simulink model "TosAeb"  (R2021a)
settings: Solver=FixedStepDiscrete  StartTime=0.0  StopTime=inf  FixedStep=0.01  RelTol=1e-3
undefined symbols (not defined in the file or in its folder): ACCModSts, AccSts1, AEB_ObjectMovingOrientation, AEB_ObjectMovingState, CN_BTCActive, CN_CurrentGearR, CN_CurrentGearR_DHT, CN_PTCActive, CN_VDCActive, CN_VSC_EspOff, CN_VSC_FCW_AEB_Error, CN_VSC_FCW_AEB_NoDispay, CN_VSC_FCW_AEB_PerfmDgdtn, CN_VSC_FCW_AEB_TempUnAvlbl, CN_VSC_VehStandstill, CNaccelAvoidMax, CNaccelAvoidMin, CNbrakePedalPress, CNcomfortStrLeftAccelMax, CNcomfortStrLeftAccelMin, CNcomfortStrRightAccelMax, CNcomfortStrRightAccelMin, CNDrvDoorOpen, CNDrvModeEco, CNDrvModeNorm, CNDrvModeOther, CNDrvModeSport, CNDrvSBRreminder, CNNull, CNobjInvalidID, CNPassDoorOpen, CNPassSBRreminder, CNPEBSAccelNegInf, CNPEBSAccelNull, CNPEBSTimeInf, CNPEBSTimeNull, CNSTOPMAX, CNSTOPNULL, CNsubjWidth, CNSwtOFF, CNSwtON, CNTTCMAX, CNTTCNULL, CNVehConfigAEBCycNone, CNVehConfigAEBNone, CNVehConfigAEBPedNone, CNVehConfigFCWNone, CNVehConfigJANone, CollisionSeverity, FALSE, FCWSnvty, JACycObjCalcInfoDefaultParams, JAPedObjCalcInfoDefaultParams, JAVehObjCalcInfoDefaultParams, latCycObjCalcInfoDefaultParams, latPedObjCalcInfoDefaultParams, longObjCalcInfoDefaultParams, ModSts, movingStatus, ObjectType ... (+1112)
libraries: simulink x3031, AEBSFunctionLib x50, Ta_Library x33, AEBSStateMachineLib x23, Mylibrary x12, simulink_extras x7, ObjSelect_lib x3, dspstat3 x2
(part 39 of 102)

== system TosAeb/FcwAebJa_Step_sys/ALG_AEBSystem/ALG_AEBController/AEB_FuncCondCalcApp/JaPedFunctionCondCalc/AEBJAPedAEBfullSuppAborCond/AEBJAPedAEBfullSuppCondCalc/AEBJAPedAEBfullSuppVehCondCalc ==
  note: Change OR to AND
  subjVel : Inport
  VehConfigIbooster : Inport  Port=2
  ESP_MasterCylindBrakePress : Inport  Port=3
  AccrPedlPosnDiagc : Inport  Port=4
  SteerWheelAngle_Deg : Inport  Port=5
  LeftTurnSWSts : Inport  Port=6
  RightTurnSWSts : Inport  Port=7
  subjLatAccel : Inport  Port=8
  VSC_JAPedAEBfullSuppCond : Inport  Port=9
  Abs : Abs
  Abs1 : Abs
  Compare To Constant1 : simulink/Logic and Bit Operations/Compare To Constant  relop===  const=FALSE  OutDataTypeStr=boolean
  Compare To Constant3 : simulink/Logic and Bit Operations/Compare To Constant  relop=<  const=P_AEB_Func_JaPedAEBfullSubjLatAccelAbsSupThres  OutDataTypeStr=boolean
  Compare To Constant4 : simulink/Logic and Bit Operations/Compare To Constant  relop===  const=TRUE  OutDataTypeStr=boolean
  Compare To Constant5 : simulink/Logic and Bit Operations/Compare To Constant  relop===  const=FALSE  OutDataTypeStr=boolean
  Compare To Constant6 : simulink/Logic and Bit Operations/Compare To Constant  relop=>  const=P_AEB_Func_JaPedAEBfullGasPedalSupThres  OutDataTypeStr=boolean
  Compare To Constant7 : simulink/Logic and Bit Operations/Compare To Constant  relop===  const=TRUE  OutDataTypeStr=boolean
  Compare To Constant8 : simulink/Logic and Bit Operations/Compare To Constant  relop=>=  const=P_AEB_Func_JaPedAEBfullSubjVelMin  OutDataTypeStr=boolean
  Compare To Constant9 : simulink/Logic and Bit Operations/Compare To Constant  relop=<=  const=P_AEB_Func_JaPedAEBfullSubjVelMax  OutDataTypeStr=boolean
  Constant : Constant  Value=P_AEB_Func_JaPedAEBfullAccrCondAborDelayTime
  Constant1 : Constant  Value=Ts
  Constant2 : Constant  Value=P_AEB_Func_JaPedAEBfullTurnSwtStsConfig
  Constant3 : Constant  Value=TRUE
  Logical Operator : Logic  AllPortsSameDT=off  OutDataTypeStr=boolean
  Logical Operator1 : Logic  Operator=OR  AllPortsSameDT=off  OutDataTypeStr=boolean
  Logical Operator2 : Logic  AllPortsSameDT=off  OutDataTypeStr=boolean
  Logical Operator3 : Logic  Operator=OR  AllPortsSameDT=off  OutDataTypeStr=boolean
  Logical Operator5 : Logic  Inputs=6  AllPortsSameDT=off  OutDataTypeStr=boolean
  Relational Operator : RelationalOperator  OutDataTypeStr=boolean  RndMeth=Simplest
  Relational Operator1 : RelationalOperator  Operator=<=  OutDataTypeStr=boolean  RndMeth=Simplest
  StateDelayAbortion1 : AEBSFunctionLib/StateDelayAbortion  SystemSampleTime=-1  VariantActivationTime=update diagram  Latency=0  AutoFrameSizeCalculation=off
  SteerWheelThresMax : Lookup_n-D  NumberOfTableDimensions=1  BreakpointsForDimension1=P_AEB_Func_JaPedAEBfullSubjVelStrWheelAngleMaxThresX  BeginIndexSearchUsingPreviousIndexResult=on  Table=P_AEB_Func_JaPedAEBfullSubjVelStrWheelAngleMaxThresY  InternalRulePriority=Speed  RndMeth=Simplest  InputSameDT=on
  SteerWheelThresMin Table : Lookup_n-D  NumberOfTableDimensions=1  BreakpointsForDimension1=P_AEB_Func_JaPedAEBfullSubjVelStrWheelAngleMinThresX  BeginIndexSearchUsingPreviousIndexResult=on  Table=P_AEB_Func_JaPedAEBfullSubjVelStrWheelAngleMinThresY  InternalRulePriority=Speed  RndMeth=Simplest  InputSameDT=on
  Switch : Switch  Criteria=u2 ~= 0
  brakeReleventSuppCond : Subsystem
  AEBJAPedAEBfullVehActiveCondFulfilled : Outport
  wires:
  VehConfigIbooster/1 -> brakeReleventSuppCond/1(VehConfigIbooster)
  Constant3/1 -> Switch/3
  Constant2/1 -> Switch/2
  Logical Operator1/1 -> Switch/1
  ESP_MasterCylindBrakePress/1 -> brakeReleventSuppCond/2(ESP_MasterCylindBrakePress)
  Compare To Constant5/1 -> Logical Operator5/2  "DriverReleventActiveCondFulfilled"
  Logical Operator3/1 -> Compare To Constant5/1
  Logical Operator2/1 -> Logical Operator5/3  "strReleventActiveCondFulfilled"
  Relational Operator/1 -> Logical Operator2/1
  SteerWheelThresMax/1 -> Relational Operator1/2
  [subjVel] -> SteerWheelThresMin Table/1, SteerWheelThresMax/1
  Relational Operator1/1 -> Logical Operator2/2
  Abs/1 -> Relational Operator/1, Relational Operator1/1
  Compare To Constant4/1 -> Logical Operator1/2
  Compare To Constant7/1 -> Logical Operator1/1
  RightTurnSWSts/1 -> Compare To Constant4/1
  Compare To Constant3/1 -> Logical Operator5/5  "LaterAccReleventActiveCondFulfilled"
  Abs1/1 -> Compare To Constant3/1
  subjLatAccel/1 -> Abs1/1
  SteerWheelThresMin Table/1 -> Relational Operator/2
  Constant1/1 -> StateDelayAbortion1/3
  Constant/1 -> StateDelayAbortion1/2
  Compare To Constant6/1 -> StateDelayAbortion1/1
  Logical Operator5/1 -> AEBJAPedAEBfullVehActiveCondFulfilled/1  "AEBJAPedAEBfullVehActiveCondFulfilled"
  subjVel/1 -> Compare To Constant8/1, [subjVel], Compare To Constant9/1
  Logical Operator/1 -> Logical Operator5/1  "VelocityReleventActiveCondFulfilled"
  Switch/1 -> Logical Operator5/4  "TrunSWReleventActiveCondFulfilled"
  AccrPedlPosnDiagc/1 -> Compare To Constant6/1
  Compare To Constant9/1 -> Logical Operator/2
  brakeReleventSuppCond/1(brakeReleventSuppCond) -> Logical Operator3/1  "BrkReleventSuppCondFulfilled"
  SteerWheelAngle_Deg/1 -> Abs/1
  Compare To Constant8/1 -> Logical Operator/1
  LeftTurnSWSts/1 -> Compare To Constant7/1
  StateDelayAbortion1/1 -> Logical Operator3/2  "accReleventSuppCondFulfilled"
  VSC_JAPedAEBfullSuppCond/1 -> Compare To Constant1/1
  Compare To Constant1/1 -> Logical Operator5/6

== system TosAeb/FcwAebJa_Step_sys/ALG_AEBSystem/ALG_AEBController/AEB_FuncCondCalcApp/JaPedFunctionCondCalc/AEBJAPedPrefillSuppAborCond/AEBJAPedPrefillAborCondCalc/AEBJAPedPrefillAborCondFinalJudge ==
  AEBJAPedPrefillObjRelAborCondFulfilled : Inport
  AEBJAPedPrefillVehRelAborCondFulfilled : Inport  Port=2
  AEBJAPedPrefillSituationRelAborCondFulfilled : Inport  Port=3
  Compare To Constant1 : simulink/Logic and Bit Operations/Compare To Constant  relop===  const=TRUE  OutDataTypeStr=boolean
  Compare To Constant2 : simulink/Logic and Bit Operations/Compare To Constant  relop===  const=TRUE  OutDataTypeStr=boolean
  Compare To Constant3 : simulink/Logic and Bit Operations/Compare To Constant  relop===  const=TRUE  OutDataTypeStr=boolean
  Logical Operator : Logic  Operator=OR  Inputs=3  AllPortsSameDT=off  OutDataTypeStr=boolean
  AEBJAPedPrefillAborCondFulfilled : Outport
  wires:
  Compare To Constant3/1 -> Logical Operator/1
  AEBJAPedPrefillVehRelAborCondFulfilled/1 -> Compare To Constant1/1
  AEBJAPedPrefillSituationRelAborCondFulfilled/1 -> Compare To Constant2/1
  Logical Operator/1 -> AEBJAPedPrefillAborCondFulfilled/1  "AEBJAPedPrefillAborCondFulfilled"
  AEBJAPedPrefillObjRelAborCondFulfilled/1 -> Compare To Constant3/1
  Compare To Constant1/1 -> Logical Operator/2
  Compare To Constant2/1 -> Logical Operator/3

== system TosAeb/FcwAebJa_Step_sys/ALG_AEBSystem/ALG_AEBController/AEB_FuncCondCalcApp/JaPedFunctionCondCalc/AEBJAPedPrefillSuppAborCond/AEBJAPedPrefillAborCondCalc/AEBJAPedPrefillObjRelAborCondCalc ==
  ObjectID : Inport
  Compare To Constant4 : simulink/Logic and Bit Operations/Compare To Constant  relop===  const=CNobjInvalidID  OutDataTypeStr=boolean
  Logical Operator : Logic  Operator=OR  AllPortsSameDT=off  OutDataTypeStr=boolean
  Relational Operator2 : RelationalOperator  Operator=~=  OutDataTypeStr=boolean  RndMeth=Simplest
  Unit Delay1 : UnitDelay  SampleTime=-1  HasFrameUpgradeWarning=on
  AEBJAPedPrefillObjRelAborCondFulfilled : Outport
  wires:
  ObjectID/1 -> Unit Delay1/1, Relational Operator2/1, Compare To Constant4/1
  Logical Operator/1 -> AEBJAPedPrefillObjRelAborCondFulfilled/1
  Unit Delay1/1 -> Relational Operator2/2
  Compare To Constant4/1 -> Logical Operator/1
  Relational Operator2/1 -> Logical Operator/2

== system TosAeb/FcwAebJa_Step_sys/ALG_AEBSystem/ALG_AEBController/AEB_FuncCondCalcApp/JaPedFunctionCondCalc/AEBJAPedPrefillSuppAborCond/AEBJAPedPrefillAborCondCalc/AEBJAPedPrefillSituationRelAborCondCalc ==
  Constant : Constant  Value=FALSE
  AEBJAPedPrefillSituationRelAborCondFulfilled : Outport
  wires:
  Constant/1 -> AEBJAPedPrefillSituationRelAborCondFulfilled/1

== system TosAeb/FcwAebJa_Step_sys/ALG_AEBSystem/ALG_AEBController/AEB_FuncCondCalcApp/JaPedFunctionCondCalc/AEBJAPedPrefillSuppAborCond/AEBJAPedPrefillAborCondCalc/AEBJAPedPrefillVehRelAborCondCalc ==
  SteerWheelSpd_DegS : Inport
  AccelPedalPositionDiag : Inport  Port=2
  VSC_JAPedPrefillAborCond : Inport  Port=3
  Abs : Abs
  Abs1 : Abs
  Compare To Constant : simulink/Logic and Bit Operations/Compare To Constant  relop=>  const=P_AEB_Func_JaPedPrefillStrWheelAngleSpdAborThres  OutDataTypeStr=boolean
  Compare To Constant1 : simulink/Logic and Bit Operations/Compare To Constant  relop=>  const=P_AEB_Func_JaPedPrefillGasPedalAborThres  OutDataTypeStr=boolean
  Compare To Constant2 : simulink/Logic and Bit Operations/Compare To Constant  relop===  const=TRUE  OutDataTypeStr=boolean
  Logical Operator2 : Logic  Operator=OR  Inputs=3  AllPortsSameDT=off  OutDataTypeStr=boolean
  AEBJAPedPrefillVehRelAborCondFulfilled : Outport
  wires:
  Compare To Constant2/1 -> Logical Operator2/3
  VSC_JAPedPrefillAborCond/1 -> Compare To Constant2/1
  Logical Operator2/1 -> AEBJAPedPrefillVehRelAborCondFulfilled/1  "AEBJAPedPrefillVehRelAborCondFulfilled"
  SteerWheelSpd_DegS/1 -> Abs/1
  Abs1/1 -> Compare To Constant1/1
  AccelPedalPositionDiag/1 -> Abs1/1
  Abs/1 -> Compare To Constant/1
  Compare To Constant/1 -> Logical Operator2/1
  Compare To Constant1/1 -> Logical Operator2/2

== system TosAeb/FcwAebJa_Step_sys/ALG_AEBSystem/ALG_AEBController/AEB_FuncCondCalcApp/JaPedFunctionCondCalc/AEBJAPedPrefillSuppAborCond/AEBJAPedPrefillSuppCondCalc/AEBJAPedPrefillSuppCondFinalJudge ==
  AEBJAPedPrefillObjRelActiveCondFulfilled : Inport
  AEBJAPedPrefillVehRelActiveCondFulfilled : Inport  Port=2
  AEBJAPedPrefillSituationRelActiveCondFulfilled : Inport  Port=3
  Compare To Constant : simulink/Logic and Bit Operations/Compare To Constant  relop===  const=FALSE  OutDataTypeStr=boolean
  Compare To Constant1 : simulink/Logic and Bit Operations/Compare To Constant  relop===  const=FALSE  OutDataTypeStr=boolean
  Compare To Constant2 : simulink/Logic and Bit Operations/Compare To Constant  relop===  const=FALSE  OutDataTypeStr=boolean
  Logical Operator : Logic  Operator=OR  Inputs=3  AllPortsSameDT=off  OutDataTypeStr=boolean
  AEBJAPedPrefillSuppCondFulfilled : Outport
  wires:
  Compare To Constant2/1 -> Logical Operator/3
  Compare To Constant1/1 -> Logical Operator/2
  Compare To Constant/1 -> Logical Operator/1
  AEBJAPedPrefillVehRelActiveCondFulfilled/1 -> Compare To Constant1/1
  AEBJAPedPrefillObjRelActiveCondFulfilled/1 -> Compare To Constant/1
  AEBJAPedPrefillSituationRelActiveCondFulfilled/1 -> Compare To Constant2/1
  Logical Operator/1 -> AEBJAPedPrefillSuppCondFulfilled/1

== system TosAeb/FcwAebJa_Step_sys/ALG_AEBSystem/ALG_AEBController/AEB_FuncCondCalcApp/JaPedFunctionCondCalc/AEBJAPedPrefillSuppAborCond/AEBJAPedPrefillSuppCondCalc/JaPedPrefillSuppSituationCondCalc ==
  distBraking : Inport
  distRadical2Collsion : Inport  Port=2
  tPredictTime : Inport  Port=3
  TTB : Inport  Port=4
  ObjColliFlg : Inport  Port=5
  Add : Sum  Inputs=2
  Add1 : Sum  Inputs=2
  Compare To Constant : simulink/Logic and Bit Operations/Compare To Constant  relop===  const=TRUE  OutDataTypeStr=boolean
  Constant2 : Constant  Value=P_AEB_Func_JaPedPrefillBrkDistSecure
  Constant3 : Constant  Value=P_AEB_Func_JaPedPrefillTtcTimeOffset
  Logical Operator1 : Logic  AllPortsSameDT=off  OutDataTypeStr=boolean
  Relational Operator : RelationalOperator  Operator=>  OutDataTypeStr=boolean  RndMeth=Simplest
  Relational Operator1 : RelationalOperator  Operator=<  OutDataTypeStr=boolean  RndMeth=Simplest
  Terminator : Terminator
  AEBJAVehPrefillSuppSitActiveCondFulfilled : Outport
  wires:
  TTB/1 -> Add1/1
  tPredictTime/1 -> Relational Operator1/1
  distRadical2Collsion/1 -> Relational Operator/2
  Add/1 -> Relational Operator/1
  distBraking/1 -> Add/1
  Constant2/1 -> Add/2
  Relational Operator1/1 -> Logical Operator1/1
  Logical Operator1/1 -> AEBJAVehPrefillSuppSitActiveCondFulfilled/1
  Relational Operator/1 -> Terminator/1
  Constant3/1 -> Add1/2
  Add1/1 -> Relational Operator1/2
  ObjColliFlg/1 -> Compare To Constant/1
  Compare To Constant/1 -> Logical Operator1/2

== system TosAeb/FcwAebJa_Step_sys/ALG_AEBSystem/ALG_AEBController/AEB_FuncCondCalcApp/JaPedFunctionCondCalc/AEBJAPedPrefillSuppAborCond/AEBJAPedPrefillSuppCondCalc/JaPedPrefillSuppSubjCondCalcu ==
  note: Chang OR to AND
  note: Change < to <=,change > to >=
  subjVel : Inport
  VehConfigIbooster : Inport  Port=2
  BrakePedalStatus_G : Inport  Port=3
  ESP_MasterCylindBrakePress : Inport  Port=4
  IB_BrkPedalAppld : Inport  Port=5
  AccrPedlPosnDiagc : Inport  Port=6
  SteerWheelAngle_Deg : Inport  Port=7
  LeftTurnSWSts : Inport  Port=8
  RightTurnSWSts : Inport  Port=9
  subjLatAccel : Inport  Port=10
  VSC_JAPedPrefillSuppCond : Inport  Port=11
  1-D Lookup Table : Lookup_n-D  NumberOfTableDimensions=1  BreakpointsForDimension1=P_AEB_Func_JaPedPrefillSubjVelStrWheelAngleMinThresX  BeginIndexSearchUsingPreviousIndexResult=on  Table=P_AEB_Func_JaPedPrefillSubjVelStrWheelAngleMinThresY  ExtrapMethod=Clip  InternalRulePriority=Speed  RndMeth=Simplest  InputSameDT=on
  1-D Lookup Table1 : Lookup_n-D  NumberOfTableDimensions=1  BreakpointsForDimension1=P_AEB_Func_JaPedPrefillSubjVelStrWheelAngleMaxThresX  BeginIndexSearchUsingPreviousIndexResult=on  Table=P_AEB_Func_JaPedPrefillSubjVelStrWheelAngleMaxThresY  ExtrapMethod=Clip  InternalRulePriority=Speed  RndMeth=Simplest  InputSameDT=on
  Abs : Abs
  Abs1 : Abs
  Compare To Constant2 : simulink/Logic and Bit Operations/Compare To Constant  relop===  const=FALSE  OutDataTypeStr=boolean
  Compare To Constant3 : simulink/Logic and Bit Operations/Compare To Constant  relop=<  const=P_AEB_Func_JaPedPrefillSubjLatAccelAbsSupThres  OutDataTypeStr=boolean
  Compare To Constant4 : simulink/Logic and Bit Operations/Compare To Constant  relop===  const=FALSE  OutDataTypeStr=boolean
  Compare To Constant5 : simulink/Logic and Bit Operations/Compare To Constant  relop===  const=TRUE  OutDataTypeStr=boolean
  Compare To Constant6 : simulink/Logic and Bit Operations/Compare To Constant  relop=>  const=P_AEB_Func_JaPedPrefillGasPedalSupThres  OutDataTypeStr=boolean
  Compare To Constant7 : simulink/Logic and Bit Operations/Compare To Constant  relop===  const=TRUE  OutDataTypeStr=boolean
  Compare To Constant8 : simulink/Logic and Bit Operations/Compare To Constant  relop=>=  const=P_AEB_Func_JaPedPrefillSubjVelMin  OutDataTypeStr=boolean
  Compare To Constant9 : simulink/Logic and Bit Operations/Compare To Constant  relop=<=  const=P_AEB_Func_JaPedPrefillSubjVelMax  OutDataTypeStr=boolean
  Constant : Constant  Value=P_AEB_Func_JaPedPrefillAccrCondAborDelayTime
  Constant1 : Constant  Value=Ts
  Constant2 : Constant  Value=P_AEB_Func_JaPedPrefillTurnSwtStsConfig
  Constant3 : Constant  Value=TRUE
  Logical Operator : Logic  AllPortsSameDT=off  OutDataTypeStr=boolean
  Logical Operator1 : Logic  Operator=OR  AllPortsSameDT=off  OutDataTypeStr=boolean
  Logical Operator2 : Logic  AllPortsSameDT=off  OutDataTypeStr=boolean
  Logical Operator4 : Logic  Operator=OR  AllPortsSameDT=off  OutDataTypeStr=boolean
  Logical Operator5 : Logic  Inputs=6  AllPortsSameDT=off  OutDataTypeStr=boolean
  Relational Operator : RelationalOperator  OutDataTypeStr=boolean  RndMeth=Simplest
  Relational Operator1 : RelationalOperator  Operator=<=  OutDataTypeStr=boolean  RndMeth=Simplest
  StateDelayAbortion : AEBSFunctionLib/StateDelayAbortion  SystemSampleTime=-1  VariantActivationTime=update diagram  Latency=0  AutoFrameSizeCalculation=off
  Switch : Switch  Criteria=u2 ~= 0
  brakeReleventSuppCond : Subsystem
  AEBJAPedPrefillVehActiveCondFulfilled : Outport
  wires:
  BrakePedalStatus_G/1 -> brakeReleventSuppCond/2(BrakePedalStatus_G)
  ESP_MasterCylindBrakePress/1 -> brakeReleventSuppCond/3(ESP_MasterCylindBrakePress)
  VehConfigIbooster/1 -> brakeReleventSuppCond/1(VehConfigIbooster)
  Logical Operator1/1 -> Switch/1
  Constant2/1 -> Switch/2
  Constant3/1 -> Switch/3
  IB_BrkPedalAppld/1 -> brakeReleventSuppCond/4(IB_BrkPedalAppld)
  Compare To Constant2/1 -> Logical Operator5/2
  Logical Operator4/1 -> Compare To Constant2/1
  Relational Operator1/1 -> Logical Operator2/2
  1-D Lookup Table1/1 -> Relational Operator1/2
  Relational Operator/1 -> Logical Operator2/1
  Compare To Constant5/1 -> Logical Operator1/2
  Compare To Constant7/1 -> Logical Operator1/1
  RightTurnSWSts/1 -> Compare To Constant5/1
  LeftTurnSWSts/1 -> Compare To Constant7/1
  Compare To Constant8/1 -> Logical Operator/1
  Logical Operator2/1 -> Logical Operator5/3  "strReleventActivepCondFulfilled"
  brakeReleventSuppCond/1(brakeReleventSuppCond) -> Logical Operator4/1  "BrkReleventSuppCondFulfilled"
  Compare To Constant9/1 -> Logical Operator/2
  AccrPedlPosnDiagc/1 -> Compare To Constant6/1
  Switch/1 -> Logical Operator5/4  "TrunSWReleventActiveCondFulfilled"
  Logical Operator/1 -> Logical Operator5/1  "VelocityReleventActiveCondFulfilled"
  subjVel/1 -> [subjVel], Compare To Constant8/1, Compare To Constant9/1
  Logical Operator5/1 -> AEBJAPedPrefillVehActiveCondFulfilled/1  "AEBJAPedPrefillVehActiveCondFulfilled"
  Constant/1 -> StateDelayAbortion/2
  Constant1/1 -> StateDelayAbortion/3
  [subjVel] -> 1-D Lookup Table/1, 1-D Lookup Table1/1
  1-D Lookup Table/1 -> Relational Operator/2
  subjLatAccel/1 -> Abs1/1
  Abs1/1 -> Compare To Constant3/1
  Compare To Constant3/1 -> Logical Operator5/5  "LaterAccReleventActiveCondFulfilled"
  VSC_JAPedPrefillSuppCond/1 -> Compare To Constant4/1
  Compare To Constant4/1 -> Logical Operator5/6  "VSCReleventActiveCondFulfilled"
  SteerWheelAngle_Deg/1 -> Abs/1
  Abs/1 -> Relational Operator1/1, Relational Operator/1
  Compare To Constant6/1 -> StateDelayAbortion/1
  StateDelayAbortion/1 -> Logical Operator4/2  "accReleventSuppCondFulfilled"

== system TosAeb/FcwAebJa_Step_sys/ALG_AEBSystem/ALG_AEBController/AEB_FuncCondCalcApp/JaPedFunctionCondCalc/AEBJAPedPrefillSuppAborCond/AEBJAPedPrefillSuppCondCalc/JaPedhPrefillSuppObjCondCalcu ==
  ObjectMovingOrientation : Inport
  ObjectID : Inport  Port=2
  ObjectExistProb : Inport  Port=3
  ObjectIsObstacleProb : Inport  Port=4
  ObjectTypeCameraConfirmed : Inport  Port=5
  dy : Inport  Port=6
  vxAbsObj : Inport  Port=7
  vyRelCorr : Inport  Port=8
  ObjFusQuality : Inport  Port=9
  Abs : Abs
  Compare To Constant1 : simulink/Logic and Bit Operations/Compare To Constant  relop=>  const=P_AEB_Func_JaPedPrefillObjVelSupThresMin  OutDataTypeStr=boolean
  Compare To Constant11 : simulink/Logic and Bit Operations/Compare To Constant  relop===  const=TRUE  OutDataTypeStr=boolean
  Compare To Constant15 : simulink/Logic and Bit Operations/Compare To Constant  relop=~=  const=CNobjInvalidID  OutDataTypeStr=boolean
  Compare To Constant16 : simulink/Logic and Bit Operations/Compare To Constant  relop=>  const=P_AEB_Func_JaPedPrefillObjObstacleProbSupThres  OutDataTypeStr=boolean
  Compare To Constant17 : simulink/Logic and Bit Operations/Compare To Constant  relop=~=  const=CNobjInvalidID  OutDataTypeStr=boolean
  Compare To Constant18 : simulink/Logic and Bit Operations/Compare To Constant  relop===  const=TRUE  OutDataTypeStr=boolean
  Compare To Constant2 : simulink/Logic and Bit Operations/Compare To Constant  relop=<  const=P_AEB_Func_JaPedPrefillObjVelSupThresMax  OutDataTypeStr=boolean
  Compare To Constant3 : simulink/Logic and Bit Operations/Compare To Constant  relop===  const=AEB_ObjectMovingOrientation.Crossing  OutDataTypeStr=boolean
  Compare To Constant4 : simulink/Logic and Bit Operations/Compare To Constant  relop===  const=AEB_ObjectMovingOrientation.InvDir  OutDataTypeStr=boolean
  Compare To Constant5 : simulink/Logic and Bit Operations/Compare To Constant  relop=<  const=P_AEB_Func_JaPedPrefillObjDySupThres  OutDataTypeStr=boolean
  Logical Operator1 : Logic  AllPortsSameDT=off  OutDataTypeStr=boolean
  Logical Operator2 : Logic  Operator=OR  AllPortsSameDT=off  OutDataTypeStr=boolean
  Logical Operator4 : Logic  Inputs=7  AllPortsSameDT=off  OutDataTypeStr=boolean
  Logical Operator6 : Logic  AllPortsSameDT=off  OutDataTypeStr=boolean
  Logical Operator7 : Logic  AllPortsSameDT=off  OutDataTypeStr=boolean
  Math Function : Math  Operator=hypot  SignedPower=on  SaturateOnIntegerOverflow=on
  Product1 : Product
  Relational Operator2 : RelationalOperator  Operator===  OutDataTypeStr=boolean  RndMeth=Simplest
  Unit Delay1 : UnitDelay  SampleTime=-1  HasFrameUpgradeWarning=on
  AEBJAPedPrefillSuppObjActiveCondFulfilled : Outport
  wires:
  Compare To Constant11/1 -> Logical Operator4/7  "ObjFusQuality"
  ObjFusQuality/1 -> Compare To Constant11/1
  Compare To Constant5/1 -> Logical Operator4/5
  Abs/1 -> Compare To Constant5/1
  dy/1 -> Abs/1
  Compare To Constant18/1 -> Logical Operator4/4  "objFusionCondFulfilled"
  Logical Operator2/1 -> Logical Operator4/1  "objMovingOrientationCondFulfilled"
  Logical Operator6/1 -> Logical Operator4/2  "objIDCondFulfilled"
  Logical Operator7/1 -> Logical Operator4/3  "objQualityCondFulfilled"
  Logical Operator1/1 -> Logical Operator4/6  "objVelocityCondFulfilled"
  Compare To Constant3/1 -> Logical Operator2/2
  Compare To Constant4/1 -> Logical Operator2/1
  ObjectMovingOrientation/1 -> Compare To Constant4/1, Compare To Constant3/1
  Compare To Constant15/1 -> Logical Operator6/1
  Relational Operator2/1 -> Logical Operator6/2
  Unit Delay1/1 -> Relational Operator2/2
  ObjectID/1 -> Compare To Constant17/1, Unit Delay1/1, Relational Operator2/1, Compare To Constant15/1
  ObjectExistProb/1 -> Product1/1
  Product1/1 -> Compare To Constant16/1
  ObjectIsObstacleProb/1 -> Product1/2
  ObjectTypeCameraConfirmed/1 -> Compare To Constant18/1
  vxAbsObj/1 -> Math Function/1
  vyRelCorr/1 -> Math Function/2
  Math Function/1 -> Compare To Constant1/1, Compare To Constant2/1
  Compare To Constant1/1 -> Logical Operator1/1
  Compare To Constant2/1 -> Logical Operator1/2
  Logical Operator4/1 -> AEBJAPedPrefillSuppObjActiveCondFulfilled/1
  Compare To Constant17/1 -> Logical Operator7/1
  Compare To Constant16/1 -> Logical Operator7/2

== system TosAeb/FcwAebJa_Step_sys/ALG_AEBSystem/ALG_AEBController/AEB_FuncCondCalcApp/JaVehFunctionCondCalc/AEBJAVehAEBfullSuppAborCond/AEBJAVehAEBfullAborCondCalc/AEBJAVehAEBfullAborCondFinalJudge ==
  AEBJAVehAEBfullObjRelAborCondFulfilled : Inport
  AEBJAVehAEBfullVehRelAborCondFulfilled : Inport  Port=2
  Compare To Constant1 : simulink/Logic and Bit Operations/Compare To Constant  relop===  const=TRUE  OutDataTypeStr=boolean
  Compare To Constant3 : simulink/Logic and Bit Operations/Compare To Constant  relop===  const=TRUE  OutDataTypeStr=boolean
  Logical Operator : Logic  Operator=OR  AllPortsSameDT=off  OutDataTypeStr=boolean
  AEBJAVehAEBfullAborCondNoStndstillFulfilled : Outport
  AEBJAVehAEBfullAborCondStndstillFulfilled : Outport  Port=2
  wires:
  Compare To Constant3/1 -> Logical Operator/1
  AEBJAVehAEBfullVehRelAborCondFulfilled/1 -> Compare To Constant1/1
  Logical Operator/1 -> AEBJAVehAEBfullAborCondNoStndstillFulfilled/1  "AEBJAVehAEBfullAborCondNoStndstillFulfilled"
  AEBJAVehAEBfullObjRelAborCondFulfilled/1 -> Compare To Constant3/1
  Compare To Constant1/1 -> Logical Operator/2, AEBJAVehAEBfullAborCondStndstillFulfilled/1  "AEBJAVehAEBfullAborCondStndstillFulfilled"

== system TosAeb/FcwAebJa_Step_sys/ALG_AEBSystem/ALG_AEBController/AEB_FuncCondCalcApp/JaVehFunctionCondCalc/AEBJAVehAEBfullSuppAborCond/AEBJAVehAEBfullAborCondCalc/AEBJAVehAEBfullObjRelAborCondCalc ==
  ObjectID : Inport
  Compare To Constant4 : simulink/Logic and Bit Operations/Compare To Constant  relop===  const=CNobjInvalidID  OutDataTypeStr=boolean
  Logical Operator : Logic  Operator=OR  AllPortsSameDT=off  OutDataTypeStr=boolean
  Relational Operator2 : RelationalOperator  Operator=~=  OutDataTypeStr=boolean  RndMeth=Simplest
  Unit Delay1 : UnitDelay  SampleTime=-1  HasFrameUpgradeWarning=on
  AEBJAVehAEBfullObjRelAborCondFulfilled : Outport
  wires:
  Logical Operator/1 -> AEBJAVehAEBfullObjRelAborCondFulfilled/1
  ObjectID/1 -> Unit Delay1/1, Relational Operator2/1, Compare To Constant4/1
  Unit Delay1/1 -> Relational Operator2/2
  Compare To Constant4/1 -> Logical Operator/1
  Relational Operator2/1 -> Logical Operator/2

== system TosAeb/FcwAebJa_Step_sys/ALG_AEBSystem/ALG_AEBController/AEB_FuncCondCalcApp/JaVehFunctionCondCalc/AEBJAVehAEBfullSuppAborCond/AEBJAVehAEBfullAborCondCalc/AEBJAVehAEBfullVehRelAborCondCalc ==
  SteerWheelAngleSign : Inport
  SteerWheelSpd_DegS : Inport  Port=2
  AccelPedalPositionDiag : Inport  Port=3
  VSC_JAVehAEBfullAborCond : Inport  Port=4
  Abs : Abs
  Abs1 : Abs
  Compare To Constant : simulink/Logic and Bit Operations/Compare To Constant  relop=>  const=P_AEB_Func_JaVehAEBfullStrWheelAngleSpdAborThres  OutDataTypeStr=boolean
  Compare To Constant1 : simulink/Logic and Bit Operations/Compare To Constant  relop=>  const=P_AEB_Func_JaVehAEBfullGasPedalAborThres  OutDataTypeStr=boolean
  Compare To Constant2 : simulink/Logic and Bit Operations/Compare To Constant  relop===  const=TRUE  OutDataTypeStr=boolean
  Compare To Constant3 : simulink/Logic and Bit Operations/Compare To Constant  relop===  const=TRUE  OutDataTypeStr=boolean
  Logical Operator2 : Logic  Operator=OR  Inputs=4  AllPortsSameDT=off  OutDataTypeStr=boolean
  AEBJAVehAEBfullVehRelAborCondFulfilled : Outport
  wires:
  Compare To Constant3/1 -> Logical Operator2/4
  VSC_JAVehAEBfullAborCond/1 -> Compare To Constant3/1
  Logical Operator2/1 -> AEBJAVehAEBfullVehRelAborCondFulfilled/1  "AEBJAVehAEBfullVehRelAborCondFulfilled"
  SteerWheelSpd_DegS/1 -> Abs/1
  Abs1/1 -> Compare To Constant1/1
  AccelPedalPositionDiag/1 -> Abs1/1
  Abs/1 -> Compare To Constant/1
  SteerWheelAngleSign/1 -> Compare To Constant2/1
  Compare To Constant2/1 -> Logical Operator2/1
  Compare To Constant/1 -> Logical Operator2/2
  Compare To Constant1/1 -> Logical Operator2/3

== system TosAeb/FcwAebJa_Step_sys/ALG_AEBSystem/ALG_AEBController/AEB_FuncCondCalcApp/JaVehFunctionCondCalc/AEBJAVehAEBfullSuppAborCond/AEBJAVehAEBfullSuppCondCalc/AEBJAVehAEBfullSuppCondFinalJudge ==
  AEBJAVehAEBfullObjRelActiveCondFulfilled : Inport
  AEBJAVehAEBfullVehRelActiveCondFulfilled : Inport  Port=2
  AEBJAVehAEBfullSituationRelActiveCondFulfilled : Inport  Port=3
  Compare To Constant : simulink/Logic and Bit Operations/Compare To Constant  relop===  const=FALSE  OutDataTypeStr=boolean
  Compare To Constant1 : simulink/Logic and Bit Operations/Compare To Constant  relop===  const=FALSE  OutDataTypeStr=boolean
  Compare To Constant2 : simulink/Logic and Bit Operations/Compare To Constant  relop===  const=FALSE  OutDataTypeStr=boolean
  Logical Operator : Logic  Operator=OR  Inputs=3  AllPortsSameDT=off  OutDataTypeStr=boolean
  AEBJAVehAEBfullSuppCondFulfilled : Outport
  wires:
  Compare To Constant2/1 -> Logical Operator/3
  Compare To Constant1/1 -> Logical Operator/2
  Compare To Constant/1 -> Logical Operator/1
  AEBJAVehAEBfullVehRelActiveCondFulfilled/1 -> Compare To Constant1/1
  AEBJAVehAEBfullObjRelActiveCondFulfilled/1 -> Compare To Constant/1
  AEBJAVehAEBfullSituationRelActiveCondFulfilled/1 -> Compare To Constant2/1
  Logical Operator/1 -> AEBJAVehAEBfullSuppCondFulfilled/1

== system TosAeb/FcwAebJa_Step_sys/ALG_AEBSystem/ALG_AEBController/AEB_FuncCondCalcApp/JaVehFunctionCondCalc/AEBJAVehAEBfullSuppAborCond/AEBJAVehAEBfullSuppCondCalc/AEBJAVehAEBfullSuppObjCondCalcu ==
  ObjectMovingState : Inport
  ObjectMovingOrientation : Inport  Port=2
  ObjectID : Inport  Port=3
  ObjectExistProb : Inport  Port=4
  ObjectIsObstacleProb : Inport  Port=5
  ObjectTypeCameraConfirmed : Inport  Port=6
  dy : Inport  Port=7
  vxAbsObj : Inport  Port=8
  vyRelCorr : Inport  Port=9
  ObjFusQuality : Inport  Port=10
  Compare To Constant1 : simulink/Logic and Bit Operations/Compare To Constant  relop=>  const=P_AEB_Func_JaVehAEBfullObjVelSupThresMin  OutDataTypeStr=boolean
  Compare To Constant11 : simulink/Logic and Bit Operations/Compare To Constant  relop===  const=TRUE  OutDataTypeStr=boolean
  Compare To Constant14 : simulink/Logic and Bit Operations/Compare To Constant  relop===  const=AEB_ObjectMovingState.Moving  OutDataTypeStr=boolean
  Compare To Constant15 : simulink/Logic and Bit Operations/Compare To Constant  relop=~=  const=CNobjInvalidID  OutDataTypeStr=boolean
  Compare To Constant16 : simulink/Logic and Bit Operations/Compare To Constant  relop=>  const=P_AEB_Func_JaVehAEBfullObjObstacleProbSupThres  OutDataTypeStr=boolean
  Compare To Constant17 : simulink/Logic and Bit Operations/Compare To Constant  relop=~=  const=CNobjInvalidID  OutDataTypeStr=boolean
  Compare To Constant18 : simulink/Logic and Bit Operations/Compare To Constant  relop===  const=TRUE  OutDataTypeStr=boolean
  Compare To Constant2 : simulink/Logic and Bit Operations/Compare To Constant  relop=<  const=P_AEB_Func_JaVehAEBfullObjVelSupThresMax  OutDataTypeStr=boolean
  Compare To Constant3 : simulink/Logic and Bit Operations/Compare To Constant  relop=>  const=P_AEB_Func_JaVehAEBfullObjDySupThresMin  OutDataTypeStr=boolean
  Compare To Constant4 : simulink/Logic and Bit Operations/Compare To Constant  relop===  const=AEB_ObjectMovingOrientation.InvDir  OutDataTypeStr=boolean
  Compare To Constant5 : simulink/Logic and Bit Operations/Compare To Constant  relop=<  const=P_AEB_Func_JaVehAEBfullObjDySupThresMax  OutDataTypeStr=boolean
  Logical Operator{1..2,6..7} (4 alike) : Logic  AllPortsSameDT=off  OutDataTypeStr=boolean
  Logical Operator4 : Logic  Inputs=8  AllPortsSameDT=off  OutDataTypeStr=boolean
  Math Function : Math  Operator=hypot  SignedPower=on  SaturateOnIntegerOverflow=on
  Product1 : Product
  Relational Operator2 : RelationalOperator  Operator===  OutDataTypeStr=boolean  RndMeth=Simplest
  Unit Delay1 : UnitDelay  SampleTime=-1  HasFrameUpgradeWarning=on
  AEBJAVehAEBfullObjActiveCondFulfilled : Outport
  wires:
  Compare To Constant11/1 -> Logical Operator4/8  "ObjFusQuality"
  ObjFusQuality/1 -> Compare To Constant11/1
  Logical Operator2/1 -> Logical Operator4/6  "objVelocityCondFulfilled"
  Compare To Constant5/1 -> Logical Operator2/2
  Compare To Constant3/1 -> Logical Operator2/1
  dy/1 -> Compare To Constant3/1, Compare To Constant5/1
  ObjectMovingState/1 -> Compare To Constant14/1
  Compare To Constant14/1 -> Logical Operator4/1  "objMovingStateCondFulfilled"
  ObjectMovingOrientation/1 -> Compare To Constant4/1
  Compare To Constant4/1 -> Logical Operator4/2  "objMovingOrientationCondFulfilled"
  Compare To Constant15/1 -> Logical Operator6/1
  Logical Operator6/1 -> Logical Operator4/3  "objIDCondFulfilled"
  Relational Operator2/1 -> Logical Operator6/2
  Unit Delay1/1 -> Relational Operator2/2
  ObjectID/1 -> Unit Delay1/1, Compare To Constant17/1, Compare To Constant15/1, Relational Operator2/1
  ObjectExistProb/1 -> Product1/1
  Product1/1 -> Compare To Constant16/1
  ObjectIsObstacleProb/1 -> Product1/2
  Compare To Constant16/1 -> Logical Operator7/2
  Compare To Constant17/1 -> Logical Operator7/1
  Logical Operator7/1 -> Logical Operator4/4  "objQualityCondFulfilled"
  ObjectTypeCameraConfirmed/1 -> Compare To Constant18/1
  Compare To Constant18/1 -> Logical Operator4/5  "objFusionCondFulfilled"
  vxAbsObj/1 -> Math Function/1
  vyRelCorr/1 -> Math Function/2
  Math Function/1 -> Compare To Constant1/1, Compare To Constant2/1
  Compare To Constant1/1 -> Logical Operator1/1
  Compare To Constant2/1 -> Logical Operator1/2
  Logical Operator1/1 -> Logical Operator4/7  "objVelocityCondFulfilled"
  Logical Operator4/1 -> AEBJAVehAEBfullObjActiveCondFulfilled/1

== system TosAeb/FcwAebJa_Step_sys/ALG_AEBSystem/ALG_AEBController/AEB_FuncCondCalcApp/JaVehFunctionCondCalc/AEBJAVehAEBfullSuppAborCond/AEBJAVehAEBfullSuppCondCalc/AEBJAVehAEBfullSuppSituationCondCalc ==
  note: change ">" to "<"
  initialLatDist : Inport
  restLatDist2Collsion : Inport  Port=2
  tPredictTime : Inport  Port=3
  TTB : Inport  Port=4
  ObjColliFlg : Inport  Port=5
  Add : Sum  Inputs=2
  Add1 : Sum  Inputs=2
  Add2 : Sum  Inputs=2
  Compare To Constant : simulink/Logic and Bit Operations/Compare To Constant  relop===  const=TRUE  OutDataTypeStr=boolean
  Constant : Constant  Value=P_Global_VehicleWidthMin_m
  Constant1 : Constant  Value=P_AEB_Func_JaVehAEBfullObjWidth
  Constant2 : Constant  Value=P_AEB_Func_JaVehAEBfullBrkDistSecure
  Constant3 : Constant  Value=P_AEB_Func_JaVehAEBfullTtcTimeOffset
  Gain : Gain  Gain=0.5
  Gain1 : Gain  Gain=0.5
  Logical Operator : Logic  AllPortsSameDT=off  OutDataTypeStr=boolean
  Logical Operator2 : Logic  AllPortsSameDT=off  OutDataTypeStr=boolean
  Relational Operator : RelationalOperator  Operator=>  OutDataTypeStr=boolean  RndMeth=Simplest
  Relational Operator1 : RelationalOperator  Operator=<=  OutDataTypeStr=boolean  RndMeth=Simplest
  Relational Operator2 : RelationalOperator  Operator=<  OutDataTypeStr=boolean  RndMeth=Simplest
  Terminator : Terminator
  AEBJAVehAEBfullActiveSituationCondFulfilled : Outport
  wires:
  Compare To Constant/1 -> Logical Operator2/2
  restLatDist2Collsion/1 -> Relational Operator1/1
  Relational Operator1/1 -> Logical Operator/2
  Relational Operator/1 -> Logical Operator/1
  Add/1 -> Add1/1
  Relational Operator2/1 -> Logical Operator2/1
  Constant3/1 -> Add2/2
  Add2/1 -> Relational Operator2/2
  ObjColliFlg/1 -> Compare To Constant/1
  tPredictTime/1 -> Relational Operator2/1
  TTB/1 -> Add2/1
  initialLatDist/1 -> Relational Operator/1
  Add1/1 -> Relational Operator/2, Relational Operator1/2
  Constant/1 -> Gain/1
  Gain/1 -> Add/1
  Constant1/1 -> Gain1/1
  Gain1/1 -> Add/2
  Constant2/1 -> Add1/2
  Logical Operator2/1 -> AEBJAVehAEBfullActiveSituationCondFulfilled/1
  Logical Operator/1 -> Terminator/1

== system TosAeb/FcwAebJa_Step_sys/ALG_AEBSystem/ALG_AEBController/AEB_FuncCondCalcApp/JaVehFunctionCondCalc/AEBJAVehAEBfullSuppAborCond/AEBJAVehAEBfullSuppCondCalc/AEBJAVehAEBfullSuppSubjCondCalcu ==
  note: Change or to and
  note: Change or to and
  subjVel : Inport
  VehConfigIbooster : Inport  Port=2
  ESP_MasterCylindBrakePress : Inport  Port=3
  AccrPedlPosnDiagc : Inport  Port=4
  SteerWheelAngle_Deg : Inport  Port=5
  LeftTurnSWSts : Inport  Port=6
  LateralAcc : Inport  Port=7
  VSC_JAVehAEBfullSuppCond : Inport  Port=8
  1-D Lookup Table : Lookup_n-D  NumberOfTableDimensions=1  BreakpointsForDimension1=P_AEB_Func_JaVehAEBfullSubjVelStrWheelAngleMinThresX  BeginIndexSearchUsingPreviousIndexResult=on  Table=P_AEB_Func_JaVehAEBfullSubjVelStrWheelAngleMinThresY  InternalRulePriority=Speed  RndMeth=Simplest  InputSameDT=on
  1-D Lookup Table1 : Lookup_n-D  NumberOfTableDimensions=1  BreakpointsForDimension1=P_AEB_Func_JaVehAEBfullSubjVelStrWheelAngleMaxThresX  BeginIndexSearchUsingPreviousIndexResult=on  Table=P_AEB_Func_JaVehAEBfullSubjVelStrWheelAngleMaxThresY  InternalRulePriority=Speed  RndMeth=Simplest  InputSameDT=on
  Abs : Abs
  Abs1 : Abs
  Compare To Constant1 : simulink/Logic and Bit Operations/Compare To Constant  relop===  const=FALSE  OutDataTypeStr=boolean
  Compare To Constant2 : simulink/Logic and Bit Operations/Compare To Constant  relop===  const=FALSE  OutDataTypeStr=boolean
  Compare To Constant3 : simulink/Logic and Bit Operations/Compare To Constant  relop=<=  const=P_AEB_Func_JaVehAEBfullSubjLatAccelAbsSupThres  OutDataTypeStr=boolean
  Compare To Constant6 : simulink/Logic and Bit Operations/Compare To Constant  relop=>  const=P_AEB_Func_JaVehAEBfullGasPedalSupThres  OutDataTypeStr=boolean
  Compare To Constant7 : simulink/Logic and Bit Operations/Compare To Constant  relop===  const=TRUE  OutDataTypeStr=boolean
  Compare To Constant8 : simulink/Logic and Bit Operations/Compare To Constant  relop=>=  const=P_AEB_Func_JaVehAEBfullSubjVelMin  OutDataTypeStr=boolean
  Compare To Constant9 : simulink/Logic and Bit Operations/Compare To Constant  relop=<=  const=P_AEB_Func_JaVehAEBfullSubjVelMax  OutDataTypeStr=boolean
  Constant : Constant  Value=P_AEB_Func_JaVehAEBfullAccrCondAborDelayTime
  Constant1 : Constant  Value=Ts
  Constant2 : Constant  Value=P_AEB_Func_JaVehAEBfullTurnSwtStsConfig
  Constant3 : Constant  Value=TRUE
  Logical Operator : Logic  AllPortsSameDT=off  OutDataTypeStr=boolean
  Logical Operator1 : Logic  Operator=OR  AllPortsSameDT=off  OutDataTypeStr=boolean
  Logical Operator4 : Logic  AllPortsSameDT=off  OutDataTypeStr=boolean
  Logical Operator5 : Logic  Inputs=6  AllPortsSameDT=off  OutDataTypeStr=boolean
  Relational Operator : RelationalOperator  Operator=>  OutDataTypeStr=boolean  RndMeth=Simplest
  Relational Operator1 : RelationalOperator  Operator=<  OutDataTypeStr=boolean  RndMeth=Simplest
  StateDelayAbortion1 : AEBSFunctionLib/StateDelayAbortion  SystemSampleTime=-1  VariantActivationTime=update diagram  Latency=0  AutoFrameSizeCalculation=off
  Switch : Switch  Criteria=u2 ~= 0
  brakeReleventSuppCond : Subsystem
  AEBJAVehAEBfullVehActiveCondFulfilled : Outport
  wires:
  VehConfigIbooster/1 -> brakeReleventSuppCond/1(VehConfigIbooster)
  ESP_MasterCylindBrakePress/1 -> brakeReleventSuppCond/2(ESP_MasterCylindBrakePress)
  Compare To Constant7/1 -> Switch/1
  Constant3/1 -> Switch/3
  Constant2/1 -> Switch/2
  Logical Operator1/1 -> Compare To Constant2/1
  Compare To Constant2/1 -> Logical Operator5/2  "DriverCondFulfiiled"
  1-D Lookup Table1/1 -> Relational Operator1/2
  Relational Operator1/1 -> Logical Operator4/1
  Compare To Constant1/1 -> Logical Operator5/6  "VSC_JAVehAEBfullActiveCond"
  VSC_JAVehAEBfullSuppCond/1 -> Compare To Constant1/1
  StateDelayAbortion1/1 -> Logical Operator1/2  "accReleventSuppCondFulfilled"
  LeftTurnSWSts/1 -> Compare To Constant7/1
  Compare To Constant8/1 -> Logical Operator/1
  Abs/1 -> Relational Operator1/1, Relational Operator/1
  SteerWheelAngle_Deg/1 -> Abs/1
  Logical Operator4/1 -> Logical Operator5/3  "strReleventActiveCondFulfilled"
  brakeReleventSuppCond/1(brakeReleventSuppCond) -> Logical Operator1/1  "BrkReleventSuppCondFulfilled"
  Compare To Constant9/1 -> Logical Operator/2
  AccrPedlPosnDiagc/1 -> Compare To Constant6/1
  Switch/1 -> Logical Operator5/4  "LeftTrunSWReleventActiveCondFulfilled"
  Logical Operator/1 -> Logical Operator5/1  "VelocityReleventActiveCondFulfilled"
  subjVel/1 -> Compare To Constant9/1, Compare To Constant8/1, [subjVel]
  Logical Operator5/1 -> AEBJAVehAEBfullVehActiveCondFulfilled/1  "AEBJAVehAEBfullVehActiveCondFulfilled"
  Compare To Constant6/1 -> StateDelayAbortion1/1
  Constant/1 -> StateDelayAbortion1/2
  Constant1/1 -> StateDelayAbortion1/3
  Relational Operator/1 -> Logical Operator4/2
  [subjVel] -> 1-D Lookup Table1/1, 1-D Lookup Table/1
  1-D Lookup Table/1 -> Relational Operator/2
  LateralAcc/1 -> Abs1/1
  Abs1/1 -> Compare To Constant3/1
  Compare To Constant3/1 -> Logical Operator5/5  "VSC_JAVehAEBfullActiveCond"

== system TosAeb/FcwAebJa_Step_sys/ALG_AEBSystem/ALG_AEBController/AEB_FuncCondCalcApp/JaVehFunctionCondCalc/AEBJAVehPrefillSuppAborCond/AEBJAVehPrefillAborCondCalc/AEBJAVehPrefillObjRelAborCondCalc ==
  ObjectID : Inport
  Compare To Constant4 : simulink/Logic and Bit Operations/Compare To Constant  relop===  const=CNobjInvalidID  OutDataTypeStr=boolean
  Logical Operator : Logic  Operator=OR  AllPortsSameDT=off  OutDataTypeStr=boolean
  Relational Operator2 : RelationalOperator  Operator=~=  OutDataTypeStr=boolean  RndMeth=Simplest
  Unit Delay1 : UnitDelay  SampleTime=-1  HasFrameUpgradeWarning=on
  AEBJAVehPrefillObjRelAborCondFulfilled : Outport
  wires:
  Relational Operator2/1 -> Logical Operator/2
  Compare To Constant4/1 -> Logical Operator/1
  Unit Delay1/1 -> Relational Operator2/2
  ObjectID/1 -> Compare To Constant4/1, Relational Operator2/1, Unit Delay1/1
  Logical Operator/1 -> AEBJAVehPrefillObjRelAborCondFulfilled/1

== system TosAeb/FcwAebJa_Step_sys/ALG_AEBSystem/ALG_AEBController/AEB_FuncCondCalcApp/JaVehFunctionCondCalc/AEBJAVehPrefillSuppAborCond/AEBJAVehPrefillAborCondCalc/AEBJAVehPrefillSituationRelAborCondCalc ==
  VSC_JAVehPrefillAborCond : Inport
  Compare To Constant : simulink/Logic and Bit Operations/Compare To Constant  relop===  const=TRUE  OutDataTypeStr=boolean
  AEBJAVehPrefillSituationRelAborCondFulfilled : Outport
  wires:
  VSC_JAVehPrefillAborCond/1 -> Compare To Constant/1
  Compare To Constant/1 -> AEBJAVehPrefillSituationRelAborCondFulfilled/1

== system TosAeb/FcwAebJa_Step_sys/ALG_AEBSystem/ALG_AEBController/AEB_FuncCondCalcApp/JaVehFunctionCondCalc/AEBJAVehPrefillSuppAborCond/AEBJAVehPrefillAborCondCalc/AEBJAVehPrefillVehRelAborCondCalc ==
  SteerWheelAngleSign : Inport
  SteerWheelSpd_degS : Inport  Port=2
  AccelPedalPositionDiag : Inport  Port=3
  Abs : Abs
  Abs1 : Abs
  Compare To Constant : simulink/Logic and Bit Operations/Compare To Constant  relop=>  const=P_AEB_Func_JaVehPrefillStrWheelAngleSpdAborThres  OutDataTypeStr=boolean
  Compare To Constant1 : simulink/Logic and Bit Operations/Compare To Constant  relop=>  const=P_AEB_Func_JaVehPrefillGasPedalAborThres  OutDataTypeStr=boolean
  Compare To Constant2 : simulink/Logic and Bit Operations/Compare To Constant  relop===  const=TRUE  OutDataTypeStr=boolean
  Logical Operator2 : Logic  Operator=OR  Inputs=3  AllPortsSameDT=off  OutDataTypeStr=boolean
  AEBJAVehPrefillVehRelAborCondFulfilled : Outport
  wires:
  Compare To Constant1/1 -> Logical Operator2/3
  Compare To Constant/1 -> Logical Operator2/2
  Compare To Constant2/1 -> Logical Operator2/1
  SteerWheelAngleSign/1 -> Compare To Constant2/1
  Abs/1 -> Compare To Constant/1
  AccelPedalPositionDiag/1 -> Abs1/1
  Abs1/1 -> Compare To Constant1/1
  SteerWheelSpd_degS/1 -> Abs/1
  Logical Operator2/1 -> AEBJAVehPrefillVehRelAborCondFulfilled/1  "AEBJAVehPrefillVehRelAborCondFulfilled"

== system TosAeb/FcwAebJa_Step_sys/ALG_AEBSystem/ALG_AEBController/AEB_FuncCondCalcApp/JaVehFunctionCondCalc/AEBJAVehPrefillSuppAborCond/AEBJAVehPrefillAborCondCalc/pedPrefillAborCondFinalJudge ==
  AEBJAVehPrefillObjRelAborCondFulfilled : Inport
  AEBJAVehPrefillVehRelAborCondFulfilled : Inport  Port=2
  AEBJAVehPrefillSituationRelAborCondFulfilled : Inport  Port=3
  Compare To Constant1 : simulink/Logic and Bit Operations/Compare To Constant  relop===  const=TRUE  OutDataTypeStr=boolean
  Compare To Constant2 : simulink/Logic and Bit Operations/Compare To Constant  relop===  const=TRUE  OutDataTypeStr=boolean
  Compare To Constant3 : simulink/Logic and Bit Operations/Compare To Constant  relop===  const=TRUE  OutDataTypeStr=boolean
  Logical Operator : Logic  Operator=OR  Inputs=3  AllPortsSameDT=off  OutDataTypeStr=boolean
  AEBJAVehPrefillAborCondFulfilled : Outport
  wires:
  Compare To Constant2/1 -> Logical Operator/3
  Compare To Constant1/1 -> Logical Operator/2
  AEBJAVehPrefillObjRelAborCondFulfilled/1 -> Compare To Constant3/1
  Logical Operator/1 -> AEBJAVehPrefillAborCondFulfilled/1  "AEBJAVehPrefillAborCondFulfilled"
  AEBJAVehPrefillSituationRelAborCondFulfilled/1 -> Compare To Constant2/1
  AEBJAVehPrefillVehRelAborCondFulfilled/1 -> Compare To Constant1/1
  Compare To Constant3/1 -> Logical Operator/1

== system TosAeb/FcwAebJa_Step_sys/ALG_AEBSystem/ALG_AEBController/AEB_FuncCondCalcApp/JaVehFunctionCondCalc/AEBJAVehPrefillSuppAborCond/AEBJAVehPrefillSuppCondCalc/AEBJAVehPrefillSuppCondFinalJudge ==
  AEBJAVehPrefillObjRelActiveCondFulfilled : Inport
  AEBJAVehPrefillVehRelActiveCondFulfilled : Inport  Port=2
  AEBJAVehPrefillSituationRelActiveCondFulfilled : Inport  Port=3
  Compare To Constant : simulink/Logic and Bit Operations/Compare To Constant  relop===  const=FALSE  OutDataTypeStr=boolean
  Compare To Constant1 : simulink/Logic and Bit Operations/Compare To Constant  relop===  const=FALSE  OutDataTypeStr=boolean
  Compare To Constant2 : simulink/Logic and Bit Operations/Compare To Constant  relop===  const=FALSE  OutDataTypeStr=boolean
  Logical Operator : Logic  Operator=OR  Inputs=3  AllPortsSameDT=off  OutDataTypeStr=boolean
  AEBJAVehPrefillSuppCondFulfilled : Outport
  wires:
  Logical Operator/1 -> AEBJAVehPrefillSuppCondFulfilled/1
  AEBJAVehPrefillSituationRelActiveCondFulfilled/1 -> Compare To Constant2/1
  AEBJAVehPrefillObjRelActiveCondFulfilled/1 -> Compare To Constant/1
  AEBJAVehPrefillVehRelActiveCondFulfilled/1 -> Compare To Constant1/1
  Compare To Constant/1 -> Logical Operator/1
  Compare To Constant1/1 -> Logical Operator/2
  Compare To Constant2/1 -> Logical Operator/3
